simulink model "sim_discrete_planar_2dof"  (R2022b)
settings: Solver=VariableStepAuto  StartTime=0.0  StopTime=T_sim  FixedStep=param_global.Ta  RelTol=1e-3
InitFcn:
  | parameters_2dof;
undefined symbols (not defined in the file): coord_xaxis_len, coord_yaxis_len, coord_zaxis_len, fx, fy, fz, init_bus_param, param_global, param_path_set_points, param_robot, param_vis, path_coord_xaxis_len, path_coord_yaxis_len, path_coord_zaxis_len, path_param, q_0, q_0_p, tau_x, tau_y, tau_z, X, Y, Z
libraries: sm_lib x1241, nesl_utility x796
(part 33 of 34)

== system sim_discrete_planar_2dof/Visualization q2/static single path visualize spline (fast)/static single path visualize spline path4/Subsystem16 ==
  Spline : sm_lib/Curves and Surfaces/Spline  GraphicAmbientColor=[0.15 0.15 0.15 1.0]  GraphicDiffuseColor=path_coord_xaxis_color  DataPoints=[0 path_coord_xaxis_len; 0 0; 0 0]'  DataPointsUnits=cm  GraphicEmissiveColor=[0.0 0.0 0.0 1.0]  EndConditionType=Natural  MarkerShape=Sphere  MarkerSize=10  GraphicType=FromGeometry  GraphicOpacity=path_coord_xaxis_opacity  GraphicShininess=75  GraphicSpecularColor=[0.5 0.5 0.5 1.0]  GraphicVisPropType=SimpleVisualProperties
  Spline1 : sm_lib/Curves and Surfaces/Spline  GraphicAmbientColor=[0.15 0.15 0.15 1.0]  GraphicDiffuseColor=path_coord_yaxis_color  DataPoints=[0 0; 0 path_coord_yaxis_len; 0 0]'  DataPointsUnits=cm  GraphicEmissiveColor=[0.0 0.0 0.0 1.0]  EndConditionType=Natural  MarkerShape=Sphere  MarkerSize=10  GraphicType=FromGeometry  GraphicOpacity=path_coord_yaxis_opacity  GraphicShininess=75  GraphicSpecularColor=[0.5 0.5 0.5 1.0]  GraphicVisPropType=SimpleVisualProperties
  Spline2 : sm_lib/Curves and Surfaces/Spline  GraphicAmbientColor=[0.15 0.15 0.15 1.0]  GraphicDiffuseColor=path_coord_zaxis_color  DataPoints=[0 0; 0 0; 0 path_coord_zaxis_len]'  DataPointsUnits=cm  GraphicEmissiveColor=[0.0 0.0 0.0 1.0]  EndConditionType=Natural  MarkerShape=Sphere  MarkerSize=10  GraphicType=FromGeometry  GraphicOpacity=path_coord_zaxis_opacity  GraphicShininess=75  GraphicSpecularColor=[0.5 0.5 0.5 1.0]  GraphicVisPropType=SimpleVisualProperties
  path4_point : sm_lib/Curves and Surfaces/Point  GraphicType=Marker  MarkerShape=Sphere  MarkerSize=path_point_size  GraphicVisPropType=SimpleVisualProperties  GraphicDiffuseColor=path_point_color  GraphicOpacity=path_point_opacity  GraphicSpecularColor=[0.5 0.5 0.5 1.0]  GraphicAmbientColor=[0.15 0.15 0.15 1.0]  GraphicEmissiveColor=[0.0 0.0 0.0 1.0]  GraphicShininess=75
  world_iiwa_joint_OriginTransform4 : sm_lib/Frames and Transforms/Rigid Transform  TranslationMethod=Cartesian  TranslationStandardOffset=0  TranslationStandardOffsetUnits=m  TranslationStandardAxis=+Z  TranslationCartesianOffset=param_path_set_points.paths_set1(17, 1:3, 4)  TranslationCartesianOffsetUnits=m  TranslationROffset=0  TranslationROffsetUnits=m  TranslationZOffset=0  TranslationZOffsetUnits=m  TranslationThetaOffset=0  TranslationThetaOffsetUnits=deg  RotationMethod=RotationMatrix  RotationAngleUnits=deg  RotationStandardAxis=+Z  RotationAngle=0.0  RotationArbitraryAxis=[0 0 1]  FollAlignAxisA=+X  BaseAlignAxisA=+Y  FollAlignAxisB=+Y  BaseAlignAxisB=+Z  RotationSequenceAxes=BaseAxes  RotationSequence=XYZ  RotationSequenceAngles=[0, 0, 0]  RotationSequenceAnglesUnits=rad  RotationMatrix=eul2rotm(param_path_set_points.paths_set1(17, 4:6, 4), 'XYZ')  Quaternion=[1 0 0 0]
  coord : PMIOPort  Side=Left
  connections:
  coord/RConn1 -- world_iiwa_joint_OriginTransform4/LConn1
  path4_point/LConn1 -- world_iiwa_joint_OriginTransform4/RConn1 -- Spline/LConn1 -- Spline2/LConn1 -- Spline1/LConn1

== system sim_discrete_planar_2dof/Visualization q2/static single path visualize spline (fast)/static single path visualize spline path4/Subsystem17 ==
  Spline : sm_lib/Curves and Surfaces/Spline  GraphicAmbientColor=[0.15 0.15 0.15 1.0]  GraphicDiffuseColor=path_coord_xaxis_color  DataPoints=[0 path_coord_xaxis_len; 0 0; 0 0]'  DataPointsUnits=cm  GraphicEmissiveColor=[0.0 0.0 0.0 1.0]  EndConditionType=Natural  MarkerShape=Sphere  MarkerSize=10  GraphicType=FromGeometry  GraphicOpacity=path_coord_xaxis_opacity  GraphicShininess=75  GraphicSpecularColor=[0.5 0.5 0.5 1.0]  GraphicVisPropType=SimpleVisualProperties
  Spline1 : sm_lib/Curves and Surfaces/Spline  GraphicAmbientColor=[0.15 0.15 0.15 1.0]  GraphicDiffuseColor=path_coord_yaxis_color  DataPoints=[0 0; 0 path_coord_yaxis_len; 0 0]'  DataPointsUnits=cm  GraphicEmissiveColor=[0.0 0.0 0.0 1.0]  EndConditionType=Natural  MarkerShape=Sphere  MarkerSize=10  GraphicType=FromGeometry  GraphicOpacity=path_coord_yaxis_opacity  GraphicShininess=75  GraphicSpecularColor=[0.5 0.5 0.5 1.0]  GraphicVisPropType=SimpleVisualProperties
  Spline2 : sm_lib/Curves and Surfaces/Spline  GraphicAmbientColor=[0.15 0.15 0.15 1.0]  GraphicDiffuseColor=path_coord_zaxis_color  DataPoints=[0 0; 0 0; 0 path_coord_zaxis_len]'  DataPointsUnits=cm  GraphicEmissiveColor=[0.0 0.0 0.0 1.0]  EndConditionType=Natural  MarkerShape=Sphere  MarkerSize=10  GraphicType=FromGeometry  GraphicOpacity=path_coord_zaxis_opacity  GraphicShininess=75  GraphicSpecularColor=[0.5 0.5 0.5 1.0]  GraphicVisPropType=SimpleVisualProperties
  path4_point : sm_lib/Curves and Surfaces/Point  GraphicType=Marker  MarkerShape=Sphere  MarkerSize=path_point_size  GraphicVisPropType=SimpleVisualProperties  GraphicDiffuseColor=path_point_color  GraphicOpacity=path_point_opacity  GraphicSpecularColor=[0.5 0.5 0.5 1.0]  GraphicAmbientColor=[0.15 0.15 0.15 1.0]  GraphicEmissiveColor=[0.0 0.0 0.0 1.0]  GraphicShininess=75
  world_iiwa_joint_OriginTransform4 : sm_lib/Frames and Transforms/Rigid Transform  TranslationMethod=Cartesian  TranslationStandardOffset=0  TranslationStandardOffsetUnits=m  TranslationStandardAxis=+Z  TranslationCartesianOffset=param_path_set_points.paths_set1(18, 1:3, 4)  TranslationCartesianOffsetUnits=m  TranslationROffset=0  TranslationROffsetUnits=m  TranslationZOffset=0  TranslationZOffsetUnits=m  TranslationThetaOffset=0  TranslationThetaOffsetUnits=deg  RotationMethod=RotationMatrix  RotationAngleUnits=deg  RotationStandardAxis=+Z  RotationAngle=0.0  RotationArbitraryAxis=[0 0 1]  FollAlignAxisA=+X  BaseAlignAxisA=+Y  FollAlignAxisB=+Y  BaseAlignAxisB=+Z  RotationSequenceAxes=BaseAxes  RotationSequence=XYZ  RotationSequenceAngles=[0, 0, 0]  RotationSequenceAnglesUnits=rad  RotationMatrix=eul2rotm(param_path_set_points.paths_set1(18, 4:6, 4), 'XYZ')  Quaternion=[1 0 0 0]
  coord : PMIOPort  Side=Left
  connections:
  Spline1/LConn1 -- Spline2/LConn1 -- Spline/LConn1 -- world_iiwa_joint_OriginTransform4/RConn1 -- path4_point/LConn1
  coord/RConn1 -- world_iiwa_joint_OriginTransform4/LConn1

== system sim_discrete_planar_2dof/Visualization q2/static single path visualize spline (fast)/static single path visualize spline path4/Subsystem18 ==
  Spline : sm_lib/Curves and Surfaces/Spline  GraphicAmbientColor=[0.15 0.15 0.15 1.0]  GraphicDiffuseColor=path_coord_xaxis_color  DataPoints=[0 path_coord_xaxis_len; 0 0; 0 0]'  DataPointsUnits=cm  GraphicEmissiveColor=[0.0 0.0 0.0 1.0]  EndConditionType=Natural  MarkerShape=Sphere  MarkerSize=10  GraphicType=FromGeometry  GraphicOpacity=path_coord_xaxis_opacity  GraphicShininess=75  GraphicSpecularColor=[0.5 0.5 0.5 1.0]  GraphicVisPropType=SimpleVisualProperties
  Spline1 : sm_lib/Curves and Surfaces/Spline  GraphicAmbientColor=[0.15 0.15 0.15 1.0]  GraphicDiffuseColor=path_coord_yaxis_color  DataPoints=[0 0; 0 path_coord_yaxis_len; 0 0]'  DataPointsUnits=cm  GraphicEmissiveColor=[0.0 0.0 0.0 1.0]  EndConditionType=Natural  MarkerShape=Sphere  MarkerSize=10  GraphicType=FromGeometry  GraphicOpacity=path_coord_yaxis_opacity  GraphicShininess=75  GraphicSpecularColor=[0.5 0.5 0.5 1.0]  GraphicVisPropType=SimpleVisualProperties
  Spline2 : sm_lib/Curves and Surfaces/Spline  GraphicAmbientColor=[0.15 0.15 0.15 1.0]  GraphicDiffuseColor=path_coord_zaxis_color  DataPoints=[0 0; 0 0; 0 path_coord_zaxis_len]'  DataPointsUnits=cm  GraphicEmissiveColor=[0.0 0.0 0.0 1.0]  EndConditionType=Natural  MarkerShape=Sphere  MarkerSize=10  GraphicType=FromGeometry  GraphicOpacity=path_coord_zaxis_opacity  GraphicShininess=75  GraphicSpecularColor=[0.5 0.5 0.5 1.0]  GraphicVisPropType=SimpleVisualProperties
  path4_point : sm_lib/Curves and Surfaces/Point  GraphicType=Marker  MarkerShape=Sphere  MarkerSize=path_point_size  GraphicVisPropType=SimpleVisualProperties  GraphicDiffuseColor=path_point_color  GraphicOpacity=path_point_opacity  GraphicSpecularColor=[0.5 0.5 0.5 1.0]  GraphicAmbientColor=[0.15 0.15 0.15 1.0]  GraphicEmissiveColor=[0.0 0.0 0.0 1.0]  GraphicShininess=75
  world_iiwa_joint_OriginTransform4 : sm_lib/Frames and Transforms/Rigid Transform  TranslationMethod=Cartesian  TranslationStandardOffset=0  TranslationStandardOffsetUnits=m  TranslationStandardAxis=+Z  TranslationCartesianOffset=param_path_set_points.paths_set1(19, 1:3, 4)  TranslationCartesianOffsetUnits=m  TranslationROffset=0  TranslationROffsetUnits=m  TranslationZOffset=0  TranslationZOffsetUnits=m  TranslationThetaOffset=0  TranslationThetaOffsetUnits=deg  RotationMethod=RotationMatrix  RotationAngleUnits=deg  RotationStandardAxis=+Z  RotationAngle=0.0  RotationArbitraryAxis=[0 0 1]  FollAlignAxisA=+X  BaseAlignAxisA=+Y  FollAlignAxisB=+Y  BaseAlignAxisB=+Z  RotationSequenceAxes=BaseAxes  RotationSequence=XYZ  RotationSequenceAngles=[0, 0, 0]  RotationSequenceAnglesUnits=rad  RotationMatrix=eul2rotm(param_path_set_points.paths_set1(19, 4:6, 4), 'XYZ')  Quaternion=[1 0 0 0]
  coord : PMIOPort  Side=Left
  connections:
  Spline1/LConn1 -- Spline2/LConn1 -- Spline/LConn1 -- world_iiwa_joint_OriginTransform4/RConn1 -- path4_point/LConn1
  coord/RConn1 -- world_iiwa_joint_OriginTransform4/LConn1

== system sim_discrete_planar_2dof/Visualization q2/static single path visualize spline (fast)/static single path visualize spline path4/Subsystem19 ==
  Spline : sm_lib/Curves and Surfaces/Spline  GraphicAmbientColor=[0.15 0.15 0.15 1.0]  GraphicDiffuseColor=path_coord_xaxis_color  DataPoints=[0 path_coord_xaxis_len; 0 0; 0 0]'  DataPointsUnits=cm  GraphicEmissiveColor=[0.0 0.0 0.0 1.0]  EndConditionType=Natural  MarkerShape=Sphere  MarkerSize=10  GraphicType=FromGeometry  GraphicOpacity=path_coord_xaxis_opacity  GraphicShininess=75  GraphicSpecularColor=[0.5 0.5 0.5 1.0]  GraphicVisPropType=SimpleVisualProperties
  Spline1 : sm_lib/Curves and Surfaces/Spline  GraphicAmbientColor=[0.15 0.15 0.15 1.0]  GraphicDiffuseColor=path_coord_yaxis_color  DataPoints=[0 0; 0 path_coord_yaxis_len; 0 0]'  DataPointsUnits=cm  GraphicEmissiveColor=[0.0 0.0 0.0 1.0]  EndConditionType=Natural  MarkerShape=Sphere  MarkerSize=10  GraphicType=FromGeometry  GraphicOpacity=path_coord_yaxis_opacity  GraphicShininess=75  GraphicSpecularColor=[0.5 0.5 0.5 1.0]  GraphicVisPropType=SimpleVisualProperties
  Spline2 : sm_lib/Curves and Surfaces/Spline  GraphicAmbientColor=[0.15 0.15 0.15 1.0]  GraphicDiffuseColor=path_coord_zaxis_color  DataPoints=[0 0; 0 0; 0 path_coord_zaxis_len]'  DataPointsUnits=cm  GraphicEmissiveColor=[0.0 0.0 0.0 1.0]  EndConditionType=Natural  MarkerShape=Sphere  MarkerSize=10  GraphicType=FromGeometry  GraphicOpacity=path_coord_zaxis_opacity  GraphicShininess=75  GraphicSpecularColor=[0.5 0.5 0.5 1.0]  GraphicVisPropType=SimpleVisualProperties
  path4_point : sm_lib/Curves and Surfaces/Point  GraphicType=Marker  MarkerShape=Sphere  MarkerSize=path_point_size  GraphicVisPropType=SimpleVisualProperties  GraphicDiffuseColor=path_point_color  GraphicOpacity=path_point_opacity  GraphicSpecularColor=[0.5 0.5 0.5 1.0]  GraphicAmbientColor=[0.15 0.15 0.15 1.0]  GraphicEmissiveColor=[0.0 0.0 0.0 1.0]  GraphicShininess=75
  world_iiwa_joint_OriginTransform4 : sm_lib/Frames and Transforms/Rigid Transform  TranslationMethod=Cartesian  TranslationStandardOffset=0  TranslationStandardOffsetUnits=m  TranslationStandardAxis=+Z  TranslationCartesianOffset=param_path_set_points.paths_set1(20, 1:3, 4)  TranslationCartesianOffsetUnits=m  TranslationROffset=0  TranslationROffsetUnits=m  TranslationZOffset=0  TranslationZOffsetUnits=m  TranslationThetaOffset=0  TranslationThetaOffsetUnits=deg  RotationMethod=RotationMatrix  RotationAngleUnits=deg  RotationStandardAxis=+Z  RotationAngle=0.0  RotationArbitraryAxis=[0 0 1]  FollAlignAxisA=+X  BaseAlignAxisA=+Y  FollAlignAxisB=+Y  BaseAlignAxisB=+Z  RotationSequenceAxes=BaseAxes  RotationSequence=XYZ  RotationSequenceAngles=[0, 0, 0]  RotationSequenceAnglesUnits=rad  RotationMatrix=eul2rotm(param_path_set_points.paths_set1(20, 4:6, 4), 'XYZ')  Quaternion=[1 0 0 0]
  coord : PMIOPort  Side=Left
  connections:
  coord/RConn1 -- world_iiwa_joint_OriginTransform4/LConn1
  path4_point/LConn1 -- world_iiwa_joint_OriginTransform4/RConn1 -- Spline/LConn1 -- Spline2/LConn1 -- Spline1/LConn1

== system sim_discrete_planar_2dof/Visualization q2/static single path visualize spline (fast)/static single path visualize spline path4/Subsystem2 ==
  Spline : sm_lib/Curves and Surfaces/Spline  GraphicAmbientColor=[0.15 0.15 0.15 1.0]  GraphicDiffuseColor=path_coord_xaxis_color  DataPoints=[0 path_coord_xaxis_len; 0 0; 0 0]'  DataPointsUnits=cm  GraphicEmissiveColor=[0.0 0.0 0.0 1.0]  EndConditionType=Natural  MarkerShape=Sphere  MarkerSize=10  GraphicType=FromGeometry  GraphicOpacity=path_coord_xaxis_opacity  GraphicShininess=75  GraphicSpecularColor=[0.5 0.5 0.5 1.0]  GraphicVisPropType=SimpleVisualProperties
  Spline1 : sm_lib/Curves and Surfaces/Spline  GraphicAmbientColor=[0.15 0.15 0.15 1.0]  GraphicDiffuseColor=path_coord_yaxis_color  DataPoints=[0 0; 0 path_coord_yaxis_len; 0 0]'  DataPointsUnits=cm  GraphicEmissiveColor=[0.0 0.0 0.0 1.0]  EndConditionType=Natural  MarkerShape=Sphere  MarkerSize=10  GraphicType=FromGeometry  GraphicOpacity=path_coord_yaxis_opacity  GraphicShininess=75  GraphicSpecularColor=[0.5 0.5 0.5 1.0]  GraphicVisPropType=SimpleVisualProperties
  Spline2 : sm_lib/Curves and Surfaces/Spline  GraphicAmbientColor=[0.15 0.15 0.15 1.0]  GraphicDiffuseColor=path_coord_zaxis_color  DataPoints=[0 0; 0 0; 0 path_coord_zaxis_len]'  DataPointsUnits=cm  GraphicEmissiveColor=[0.0 0.0 0.0 1.0]  EndConditionType=Natural  MarkerShape=Sphere  MarkerSize=10  GraphicType=FromGeometry  GraphicOpacity=path_coord_zaxis_opacity  GraphicShininess=75  GraphicSpecularColor=[0.5 0.5 0.5 1.0]  GraphicVisPropType=SimpleVisualProperties
  path4_point : sm_lib/Curves and Surfaces/Point  GraphicType=Marker  MarkerShape=Sphere  MarkerSize=path_point_size  GraphicVisPropType=SimpleVisualProperties  GraphicDiffuseColor=path_point_color  GraphicOpacity=path_point_opacity  GraphicSpecularColor=[0.5 0.5 0.5 1.0]  GraphicAmbientColor=[0.15 0.15 0.15 1.0]  GraphicEmissiveColor=[0.0 0.0 0.0 1.0]  GraphicShininess=75
  world_iiwa_joint_OriginTransform4 : sm_lib/Frames and Transforms/Rigid Transform  TranslationMethod=Cartesian  TranslationStandardOffset=0  TranslationStandardOffsetUnits=m  TranslationStandardAxis=+Z  TranslationCartesianOffset=param_path_set_points.paths_set1(3, 1:3, 4)  TranslationCartesianOffsetUnits=m  TranslationROffset=0  TranslationROffsetUnits=m  TranslationZOffset=0  TranslationZOffsetUnits=m  TranslationThetaOffset=0  TranslationThetaOffsetUnits=deg  RotationMethod=RotationMatrix  RotationAngleUnits=deg  RotationStandardAxis=+Z  RotationAngle=0.0  RotationArbitraryAxis=[0 0 1]  FollAlignAxisA=+X  BaseAlignAxisA=+Y  FollAlignAxisB=+Y  BaseAlignAxisB=+Z  RotationSequenceAxes=BaseAxes  RotationSequence=XYZ  RotationSequenceAngles=[0, 0, 0]  RotationSequenceAnglesUnits=rad  RotationMatrix=eul2rotm(param_path_set_points.paths_set1(3, 4:6, 4), 'XYZ')  Quaternion=[1 0 0 0]
  coord : PMIOPort  Side=Left
  connections:
  coord/RConn1 -- world_iiwa_joint_OriginTransform4/LConn1
  path4_point/LConn1 -- world_iiwa_joint_OriginTransform4/RConn1 -- Spline/LConn1 -- Spline2/LConn1 -- Spline1/LConn1

== system sim_discrete_planar_2dof/Visualization q2/static single path visualize spline (fast)/static single path visualize spline path4/Subsystem3 ==
  Spline : sm_lib/Curves and Surfaces/Spline  GraphicAmbientColor=[0.15 0.15 0.15 1.0]  GraphicDiffuseColor=path_coord_xaxis_color  DataPoints=[0 path_coord_xaxis_len; 0 0; 0 0]'  DataPointsUnits=cm  GraphicEmissiveColor=[0.0 0.0 0.0 1.0]  EndConditionType=Natural  MarkerShape=Sphere  MarkerSize=10  GraphicType=FromGeometry  GraphicOpacity=path_coord_xaxis_opacity  GraphicShininess=75  GraphicSpecularColor=[0.5 0.5 0.5 1.0]  GraphicVisPropType=SimpleVisualProperties
  Spline1 : sm_lib/Curves and Surfaces/Spline  GraphicAmbientColor=[0.15 0.15 0.15 1.0]  GraphicDiffuseColor=path_coord_yaxis_color  DataPoints=[0 0; 0 path_coord_yaxis_len; 0 0]'  DataPointsUnits=cm  GraphicEmissiveColor=[0.0 0.0 0.0 1.0]  EndConditionType=Natural  MarkerShape=Sphere  MarkerSize=10  GraphicType=FromGeometry  GraphicOpacity=path_coord_yaxis_opacity  GraphicShininess=75  GraphicSpecularColor=[0.5 0.5 0.5 1.0]  GraphicVisPropType=SimpleVisualProperties
  Spline2 : sm_lib/Curves and Surfaces/Spline  GraphicAmbientColor=[0.15 0.15 0.15 1.0]  GraphicDiffuseColor=path_coord_zaxis_color  DataPoints=[0 0; 0 0; 0 path_coord_zaxis_len]'  DataPointsUnits=cm  GraphicEmissiveColor=[0.0 0.0 0.0 1.0]  EndConditionType=Natural  MarkerShape=Sphere  MarkerSize=10  GraphicType=FromGeometry  GraphicOpacity=path_coord_zaxis_opacity  GraphicShininess=75  GraphicSpecularColor=[0.5 0.5 0.5 1.0]  GraphicVisPropType=SimpleVisualProperties
  path4_point : sm_lib/Curves and Surfaces/Point  GraphicType=Marker  MarkerShape=Sphere  MarkerSize=path_point_size  GraphicVisPropType=SimpleVisualProperties  GraphicDiffuseColor=path_point_color  GraphicOpacity=path_point_opacity  GraphicSpecularColor=[0.5 0.5 0.5 1.0]  GraphicAmbientColor=[0.15 0.15 0.15 1.0]  GraphicEmissiveColor=[0.0 0.0 0.0 1.0]  GraphicShininess=75
  world_iiwa_joint_OriginTransform4 : sm_lib/Frames and Transforms/Rigid Transform  TranslationMethod=Cartesian  TranslationStandardOffset=0  TranslationStandardOffsetUnits=m  TranslationStandardAxis=+Z  TranslationCartesianOffset=param_path_set_points.paths_set1(4, 1:3, 4)  TranslationCartesianOffsetUnits=m  TranslationROffset=0  TranslationROffsetUnits=m  TranslationZOffset=0  TranslationZOffsetUnits=m  TranslationThetaOffset=0  TranslationThetaOffsetUnits=deg  RotationMethod=RotationMatrix  RotationAngleUnits=deg  RotationStandardAxis=+Z  RotationAngle=0.0  RotationArbitraryAxis=[0 0 1]  FollAlignAxisA=+X  BaseAlignAxisA=+Y  FollAlignAxisB=+Y  BaseAlignAxisB=+Z  RotationSequenceAxes=BaseAxes  RotationSequence=XYZ  RotationSequenceAngles=[0, 0, 0]  RotationSequenceAnglesUnits=rad  RotationMatrix=eul2rotm(param_path_set_points.paths_set1(4, 4:6, 4), 'XYZ')  Quaternion=[1 0 0 0]
  coord : PMIOPort  Side=Left
  connections:
  Spline1/LConn1 -- Spline2/LConn1 -- Spline/LConn1 -- world_iiwa_joint_OriginTransform4/RConn1 -- path4_point/LConn1
  coord/RConn1 -- world_iiwa_joint_OriginTransform4/LConn1

== system sim_discrete_planar_2dof/Visualization q2/static single path visualize spline (fast)/static single path visualize spline path4/Subsystem4 ==
  Spline : sm_lib/Curves and Surfaces/Spline  GraphicAmbientColor=[0.15 0.15 0.15 1.0]  GraphicDiffuseColor=path_coord_xaxis_color  DataPoints=[0 path_coord_xaxis_len; 0 0; 0 0]'  DataPointsUnits=cm  GraphicEmissiveColor=[0.0 0.0 0.0 1.0]  EndConditionType=Natural  MarkerShape=Sphere  MarkerSize=10  GraphicType=FromGeometry  GraphicOpacity=path_coord_xaxis_opacity  GraphicShininess=75  GraphicSpecularColor=[0.5 0.5 0.5 1.0]  GraphicVisPropType=SimpleVisualProperties
  Spline1 : sm_lib/Curves and Surfaces/Spline  GraphicAmbientColor=[0.15 0.15 0.15 1.0]  GraphicDiffuseColor=path_coord_yaxis_color  DataPoints=[0 0; 0 path_coord_yaxis_len; 0 0]'  DataPointsUnits=cm  GraphicEmissiveColor=[0.0 0.0 0.0 1.0]  EndConditionType=Natural  MarkerShape=Sphere  MarkerSize=10  GraphicType=FromGeometry  GraphicOpacity=path_coord_yaxis_opacity  GraphicShininess=75  GraphicSpecularColor=[0.5 0.5 0.5 1.0]  GraphicVisPropType=SimpleVisualProperties
  Spline2 : sm_lib/Curves and Surfaces/Spline  GraphicAmbientColor=[0.15 0.15 0.15 1.0]  GraphicDiffuseColor=path_coord_zaxis_color  DataPoints=[0 0; 0 0; 0 path_coord_zaxis_len]'  DataPointsUnits=cm  GraphicEmissiveColor=[0.0 0.0 0.0 1.0]  EndConditionType=Natural  MarkerShape=Sphere  MarkerSize=10  GraphicType=FromGeometry  GraphicOpacity=path_coord_zaxis_opacity  GraphicShininess=75  GraphicSpecularColor=[0.5 0.5 0.5 1.0]  GraphicVisPropType=SimpleVisualProperties
  path4_point : sm_lib/Curves and Surfaces/Point  GraphicType=Marker  MarkerShape=Sphere  MarkerSize=path_point_size  GraphicVisPropType=SimpleVisualProperties  GraphicDiffuseColor=path_point_color  GraphicOpacity=path_point_opacity  GraphicSpecularColor=[0.5 0.5 0.5 1.0]  GraphicAmbientColor=[0.15 0.15 0.15 1.0]  GraphicEmissiveColor=[0.0 0.0 0.0 1.0]  GraphicShininess=75
  world_iiwa_joint_OriginTransform4 : sm_lib/Frames and Transforms/Rigid Transform  TranslationMethod=Cartesian  TranslationStandardOffset=0  TranslationStandardOffsetUnits=m  TranslationStandardAxis=+Z  TranslationCartesianOffset=param_path_set_points.paths_set1(5, 1:3, 4)  TranslationCartesianOffsetUnits=m  TranslationROffset=0  TranslationROffsetUnits=m  TranslationZOffset=0  TranslationZOffsetUnits=m  TranslationThetaOffset=0  TranslationThetaOffsetUnits=deg  RotationMethod=RotationMatrix  RotationAngleUnits=deg  RotationStandardAxis=+Z  RotationAngle=0.0  RotationArbitraryAxis=[0 0 1]  FollAlignAxisA=+X  BaseAlignAxisA=+Y  FollAlignAxisB=+Y  BaseAlignAxisB=+Z  RotationSequenceAxes=BaseAxes  RotationSequence=XYZ  RotationSequenceAngles=[0, 0, 0]  RotationSequenceAnglesUnits=rad  RotationMatrix=eul2rotm(param_path_set_points.paths_set1(5, 4:6, 4), 'XYZ')  Quaternion=[1 0 0 0]
  coord : PMIOPort  Side=Left
  connections:
  coord/RConn1 -- world_iiwa_joint_OriginTransform4/LConn1
  path4_point/LConn1 -- world_iiwa_joint_OriginTransform4/RConn1 -- Spline/LConn1 -- Spline2/LConn1 -- Spline1/LConn1

== system sim_discrete_planar_2dof/Visualization q2/static single path visualize spline (fast)/static single path visualize spline path4/Subsystem5 ==
  Spline : sm_lib/Curves and Surfaces/Spline  GraphicAmbientColor=[0.15 0.15 0.15 1.0]  GraphicDiffuseColor=path_coord_xaxis_color  DataPoints=[0 path_coord_xaxis_len; 0 0; 0 0]'  DataPointsUnits=cm  GraphicEmissiveColor=[0.0 0.0 0.0 1.0]  EndConditionType=Natural  MarkerShape=Sphere  MarkerSize=10  GraphicType=FromGeometry  GraphicOpacity=path_coord_xaxis_opacity  GraphicShininess=75  GraphicSpecularColor=[0.5 0.5 0.5 1.0]  GraphicVisPropType=SimpleVisualProperties
  Spline1 : sm_lib/Curves and Surfaces/Spline  GraphicAmbientColor=[0.15 0.15 0.15 1.0]  GraphicDiffuseColor=path_coord_yaxis_color  DataPoints=[0 0; 0 path_coord_yaxis_len; 0 0]'  DataPointsUnits=cm  GraphicEmissiveColor=[0.0 0.0 0.0 1.0]  EndConditionType=Natural  MarkerShape=Sphere  MarkerSize=10  GraphicType=FromGeometry  GraphicOpacity=path_coord_yaxis_opacity  GraphicShininess=75  GraphicSpecularColor=[0.5 0.5 0.5 1.0]  GraphicVisPropType=SimpleVisualProperties
  Spline2 : sm_lib/Curves and Surfaces/Spline  GraphicAmbientColor=[0.15 0.15 0.15 1.0]  GraphicDiffuseColor=path_coord_zaxis_color  DataPoints=[0 0; 0 0; 0 path_coord_zaxis_len]'  DataPointsUnits=cm  GraphicEmissiveColor=[0.0 0.0 0.0 1.0]  EndConditionType=Natural  MarkerShape=Sphere  MarkerSize=10  GraphicType=FromGeometry  GraphicOpacity=path_coord_zaxis_opacity  GraphicShininess=75  GraphicSpecularColor=[0.5 0.5 0.5 1.0]  GraphicVisPropType=SimpleVisualProperties
  path4_point : sm_lib/Curves and Surfaces/Point  GraphicType=Marker  MarkerShape=Sphere  MarkerSize=path_point_size  GraphicVisPropType=SimpleVisualProperties  GraphicDiffuseColor=path_point_color  GraphicOpacity=path_point_opacity  GraphicSpecularColor=[0.5 0.5 0.5 1.0]  GraphicAmbientColor=[0.15 0.15 0.15 1.0]  GraphicEmissiveColor=[0.0 0.0 0.0 1.0]  GraphicShininess=75
  world_iiwa_joint_OriginTransform4 : sm_lib/Frames and Transforms/Rigid Transform  TranslationMethod=Cartesian  TranslationStandardOffset=0  TranslationStandardOffsetUnits=m  TranslationStandardAxis=+Z  TranslationCartesianOffset=param_path_set_points.paths_set1(6, 1:3, 4)  TranslationCartesianOffsetUnits=m  TranslationROffset=0  TranslationROffsetUnits=m  TranslationZOffset=0  TranslationZOffsetUnits=m  TranslationThetaOffset=0  TranslationThetaOffsetUnits=deg  RotationMethod=RotationMatrix  RotationAngleUnits=deg  RotationStandardAxis=+Z  RotationAngle=0.0  RotationArbitraryAxis=[0 0 1]  FollAlignAxisA=+X  BaseAlignAxisA=+Y  FollAlignAxisB=+Y  BaseAlignAxisB=+Z  RotationSequenceAxes=BaseAxes  RotationSequence=XYZ  RotationSequenceAngles=[0, 0, 0]  RotationSequenceAnglesUnits=rad  RotationMatrix=eul2rotm(param_path_set_points.paths_set1(6, 4:6, 4), 'XYZ')  Quaternion=[1 0 0 0]
  coord : PMIOPort  Side=Left
  connections:
  Spline1/LConn1 -- Spline2/LConn1 -- Spline/LConn1 -- world_iiwa_joint_OriginTransform4/RConn1 -- path4_point/LConn1
  coord/RConn1 -- world_iiwa_joint_OriginTransform4/LConn1

== system sim_discrete_planar_2dof/Visualization q2/static single path visualize spline (fast)/static single path visualize spline path4/Subsystem6 ==
  Spline : sm_lib/Curves and Surfaces/Spline  GraphicAmbientColor=[0.15 0.15 0.15 1.0]  GraphicDiffuseColor=path_coord_xaxis_color  DataPoints=[0 path_coord_xaxis_len; 0 0; 0 0]'  DataPointsUnits=cm  GraphicEmissiveColor=[0.0 0.0 0.0 1.0]  EndConditionType=Natural  MarkerShape=Sphere  MarkerSize=10  GraphicType=FromGeometry  GraphicOpacity=path_coord_xaxis_opacity  GraphicShininess=75  GraphicSpecularColor=[0.5 0.5 0.5 1.0]  GraphicVisPropType=SimpleVisualProperties
  Spline1 : sm_lib/Curves and Surfaces/Spline  GraphicAmbientColor=[0.15 0.15 0.15 1.0]  GraphicDiffuseColor=path_coord_yaxis_color  DataPoints=[0 0; 0 path_coord_yaxis_len; 0 0]'  DataPointsUnits=cm  GraphicEmissiveColor=[0.0 0.0 0.0 1.0]  EndConditionType=Natural  MarkerShape=Sphere  MarkerSize=10  GraphicType=FromGeometry  GraphicOpacity=path_coord_yaxis_opacity  GraphicShininess=75  GraphicSpecularColor=[0.5 0.5 0.5 1.0]  GraphicVisPropType=SimpleVisualProperties
  Spline2 : sm_lib/Curves and Surfaces/Spline  GraphicAmbientColor=[0.15 0.15 0.15 1.0]  GraphicDiffuseColor=path_coord_zaxis_color  DataPoints=[0 0; 0 0; 0 path_coord_zaxis_len]'  DataPointsUnits=cm  GraphicEmissiveColor=[0.0 0.0 0.0 1.0]  EndConditionType=Natural  MarkerShape=Sphere  MarkerSize=10  GraphicType=FromGeometry  GraphicOpacity=path_coord_zaxis_opacity  GraphicShininess=75  GraphicSpecularColor=[0.5 0.5 0.5 1.0]  GraphicVisPropType=SimpleVisualProperties
  path4_point : sm_lib/Curves and Surfaces/Point  GraphicType=Marker  MarkerShape=Sphere  MarkerSize=path_point_size  GraphicVisPropType=SimpleVisualProperties  GraphicDiffuseColor=path_point_color  GraphicOpacity=path_point_opacity  GraphicSpecularColor=[0.5 0.5 0.5 1.0]  GraphicAmbientColor=[0.15 0.15 0.15 1.0]  GraphicEmissiveColor=[0.0 0.0 0.0 1.0]  GraphicShininess=75
  world_iiwa_joint_OriginTransform4 : sm_lib/Frames and Transforms/Rigid Transform  TranslationMethod=Cartesian  TranslationStandardOffset=0  TranslationStandardOffsetUnits=m  TranslationStandardAxis=+Z  TranslationCartesianOffset=param_path_set_points.paths_set1(7, 1:3, 4)  TranslationCartesianOffsetUnits=m  TranslationROffset=0  TranslationROffsetUnits=m  TranslationZOffset=0  TranslationZOffsetUnits=m  TranslationThetaOffset=0  TranslationThetaOffsetUnits=deg  RotationMethod=RotationMatrix  RotationAngleUnits=deg  RotationStandardAxis=+Z  RotationAngle=0.0  RotationArbitraryAxis=[0 0 1]  FollAlignAxisA=+X  BaseAlignAxisA=+Y  FollAlignAxisB=+Y  BaseAlignAxisB=+Z  RotationSequenceAxes=BaseAxes  RotationSequence=XYZ  RotationSequenceAngles=[0, 0, 0]  RotationSequenceAnglesUnits=rad  RotationMatrix=eul2rotm(param_path_set_points.paths_set1(7, 4:6, 4), 'XYZ')  Quaternion=[1 0 0 0]
  coord : PMIOPort  Side=Left
  connections:
  Spline1/LConn1 -- Spline2/LConn1 -- Spline/LConn1 -- world_iiwa_joint_OriginTransform4/RConn1 -- path4_point/LConn1
  coord/RConn1 -- world_iiwa_joint_OriginTransform4/LConn1

== system sim_discrete_planar_2dof/Visualization q2/static single path visualize spline (fast)/static single path visualize spline path4/Subsystem7 ==
  Spline : sm_lib/Curves and Surfaces/Spline  GraphicAmbientColor=[0.15 0.15 0.15 1.0]  GraphicDiffuseColor=path_coord_xaxis_color  DataPoints=[0 path_coord_xaxis_len; 0 0; 0 0]'  DataPointsUnits=cm  GraphicEmissiveColor=[0.0 0.0 0.0 1.0]  EndConditionType=Natural  MarkerShape=Sphere  MarkerSize=10  GraphicType=FromGeometry  GraphicOpacity=path_coord_xaxis_opacity  GraphicShininess=75  GraphicSpecularColor=[0.5 0.5 0.5 1.0]  GraphicVisPropType=SimpleVisualProperties
  Spline1 : sm_lib/Curves and Surfaces/Spline  GraphicAmbientColor=[0.15 0.15 0.15 1.0]  GraphicDiffuseColor=path_coord_yaxis_color  DataPoints=[0 0; 0 path_coord_yaxis_len; 0 0]'  DataPointsUnits=cm  GraphicEmissiveColor=[0.0 0.0 0.0 1.0]  EndConditionType=Natural  MarkerShape=Sphere  MarkerSize=10  GraphicType=FromGeometry  GraphicOpacity=path_coord_yaxis_opacity  GraphicShininess=75  GraphicSpecularColor=[0.5 0.5 0.5 1.0]  GraphicVisPropType=SimpleVisualProperties
  Spline2 : sm_lib/Curves and Surfaces/Spline  GraphicAmbientColor=[0.15 0.15 0.15 1.0]  GraphicDiffuseColor=path_coord_zaxis_color  DataPoints=[0 0; 0 0; 0 path_coord_zaxis_len]'  DataPointsUnits=cm  GraphicEmissiveColor=[0.0 0.0 0.0 1.0]  EndConditionType=Natural  MarkerShape=Sphere  MarkerSize=10  GraphicType=FromGeometry  GraphicOpacity=path_coord_zaxis_opacity  GraphicShininess=75  GraphicSpecularColor=[0.5 0.5 0.5 1.0]  GraphicVisPropType=SimpleVisualProperties
  path4_point : sm_lib/Curves and Surfaces/Point  GraphicType=Marker  MarkerShape=Sphere  MarkerSize=path_point_size  GraphicVisPropType=SimpleVisualProperties  GraphicDiffuseColor=path_point_color  GraphicOpacity=path_point_opacity  GraphicSpecularColor=[0.5 0.5 0.5 1.0]  GraphicAmbientColor=[0.15 0.15 0.15 1.0]  GraphicEmissiveColor=[0.0 0.0 0.0 1.0]  GraphicShininess=75
  world_iiwa_joint_OriginTransform4 : sm_lib/Frames and Transforms/Rigid Transform  TranslationMethod=Cartesian  TranslationStandardOffset=0  TranslationStandardOffsetUnits=m  TranslationStandardAxis=+Z  TranslationCartesianOffset=param_path_set_points.paths_set1(8, 1:3, 4)  TranslationCartesianOffsetUnits=m  TranslationROffset=0  TranslationROffsetUnits=m  TranslationZOffset=0  TranslationZOffsetUnits=m  TranslationThetaOffset=0  TranslationThetaOffsetUnits=deg  RotationMethod=RotationMatrix  RotationAngleUnits=deg  RotationStandardAxis=+Z  RotationAngle=0.0  RotationArbitraryAxis=[0 0 1]  FollAlignAxisA=+X  BaseAlignAxisA=+Y  FollAlignAxisB=+Y  BaseAlignAxisB=+Z  RotationSequenceAxes=BaseAxes  RotationSequence=XYZ  RotationSequenceAngles=[0, 0, 0]  RotationSequenceAnglesUnits=rad  RotationMatrix=eul2rotm(param_path_set_points.paths_set1(8, 4:6, 4), 'XYZ')  Quaternion=[1 0 0 0]
  coord : PMIOPort  Side=Left
  connections:
  coord/RConn1 -- world_iiwa_joint_OriginTransform4/LConn1
  path4_point/LConn1 -- world_iiwa_joint_OriginTransform4/RConn1 -- Spline/LConn1 -- Spline2/LConn1 -- Spline1/LConn1

== system sim_discrete_planar_2dof/Visualization q2/static single path visualize spline (fast)/static single path visualize spline path4/Subsystem8 ==
  Spline : sm_lib/Curves and Surfaces/Spline  GraphicAmbientColor=[0.15 0.15 0.15 1.0]  GraphicDiffuseColor=path_coord_xaxis_color  DataPoints=[0 path_coord_xaxis_len; 0 0; 0 0]'  DataPointsUnits=cm  GraphicEmissiveColor=[0.0 0.0 0.0 1.0]  EndConditionType=Natural  MarkerShape=Sphere  MarkerSize=10  GraphicType=FromGeometry  GraphicOpacity=path_coord_xaxis_opacity  GraphicShininess=75  GraphicSpecularColor=[0.5 0.5 0.5 1.0]  GraphicVisPropType=SimpleVisualProperties
  Spline1 : sm_lib/Curves and Surfaces/Spline  GraphicAmbientColor=[0.15 0.15 0.15 1.0]  GraphicDiffuseColor=path_coord_yaxis_color  DataPoints=[0 0; 0 path_coord_yaxis_len; 0 0]'  DataPointsUnits=cm  GraphicEmissiveColor=[0.0 0.0 0.0 1.0]  EndConditionType=Natural  MarkerShape=Sphere  MarkerSize=10  GraphicType=FromGeometry  GraphicOpacity=path_coord_yaxis_opacity  GraphicShininess=75  GraphicSpecularColor=[0.5 0.5 0.5 1.0]  GraphicVisPropType=SimpleVisualProperties
  Spline2 : sm_lib/Curves and Surfaces/Spline  GraphicAmbientColor=[0.15 0.15 0.15 1.0]  GraphicDiffuseColor=path_coord_zaxis_color  DataPoints=[0 0; 0 0; 0 path_coord_zaxis_len]'  DataPointsUnits=cm  GraphicEmissiveColor=[0.0 0.0 0.0 1.0]  EndConditionType=Natural  MarkerShape=Sphere  MarkerSize=10  GraphicType=FromGeometry  GraphicOpacity=path_coord_zaxis_opacity  GraphicShininess=75  GraphicSpecularColor=[0.5 0.5 0.5 1.0]  GraphicVisPropType=SimpleVisualProperties
  path4_point : sm_lib/Curves and Surfaces/Point  GraphicType=Marker  MarkerShape=Sphere  MarkerSize=path_point_size  GraphicVisPropType=SimpleVisualProperties  GraphicDiffuseColor=path_point_color  GraphicOpacity=path_point_opacity  GraphicSpecularColor=[0.5 0.5 0.5 1.0]  GraphicAmbientColor=[0.15 0.15 0.15 1.0]  GraphicEmissiveColor=[0.0 0.0 0.0 1.0]  GraphicShininess=75
  world_iiwa_joint_OriginTransform4 : sm_lib/Frames and Transforms/Rigid Transform  TranslationMethod=Cartesian  TranslationStandardOffset=0  TranslationStandardOffsetUnits=m  TranslationStandardAxis=+Z  TranslationCartesianOffset=param_path_set_points.paths_set1(9, 1:3, 4)  TranslationCartesianOffsetUnits=m  TranslationROffset=0  TranslationROffsetUnits=m  TranslationZOffset=0  TranslationZOffsetUnits=m  TranslationThetaOffset=0  TranslationThetaOffsetUnits=deg  RotationMethod=RotationMatrix  RotationAngleUnits=deg  RotationStandardAxis=+Z  RotationAngle=0.0  RotationArbitraryAxis=[0 0 1]  FollAlignAxisA=+X  BaseAlignAxisA=+Y  FollAlignAxisB=+Y  BaseAlignAxisB=+Z  RotationSequenceAxes=BaseAxes  RotationSequence=XYZ  RotationSequenceAngles=[0, 0, 0]  RotationSequenceAnglesUnits=rad  RotationMatrix=eul2rotm(param_path_set_points.paths_set1(9, 4:6, 4), 'XYZ')  Quaternion=[1 0 0 0]
  coord : PMIOPort  Side=Left
  connections:
  coord/RConn1 -- world_iiwa_joint_OriginTransform4/LConn1
  path4_point/LConn1 -- world_iiwa_joint_OriginTransform4/RConn1 -- Spline/LConn1 -- Spline2/LConn1 -- Spline1/LConn1

== system sim_discrete_planar_2dof/Visualization q2/static single path visualize spline (fast)/static single path visualize spline path4/Subsystem9 ==
  Spline : sm_lib/Curves and Surfaces/Spline  GraphicAmbientColor=[0.15 0.15 0.15 1.0]  GraphicDiffuseColor=path_coord_xaxis_color  DataPoints=[0 path_coord_xaxis_len; 0 0; 0 0]'  DataPointsUnits=cm  GraphicEmissiveColor=[0.0 0.0 0.0 1.0]  EndConditionType=Natural  MarkerShape=Sphere  MarkerSize=10  GraphicType=FromGeometry  GraphicOpacity=path_coord_xaxis_opacity  GraphicShininess=75  GraphicSpecularColor=[0.5 0.5 0.5 1.0]  GraphicVisPropType=SimpleVisualProperties
  Spline1 : sm_lib/Curves and Surfaces/Spline  GraphicAmbientColor=[0.15 0.15 0.15 1.0]  GraphicDiffuseColor=path_coord_yaxis_color  DataPoints=[0 0; 0 path_coord_yaxis_len; 0 0]'  DataPointsUnits=cm  GraphicEmissiveColor=[0.0 0.0 0.0 1.0]  EndConditionType=Natural  MarkerShape=Sphere  MarkerSize=10  GraphicType=FromGeometry  GraphicOpacity=path_coord_yaxis_opacity  GraphicShininess=75  GraphicSpecularColor=[0.5 0.5 0.5 1.0]  GraphicVisPropType=SimpleVisualProperties
  Spline2 : sm_lib/Curves and Surfaces/Spline  GraphicAmbientColor=[0.15 0.15 0.15 1.0]  GraphicDiffuseColor=path_coord_zaxis_color  DataPoints=[0 0; 0 0; 0 path_coord_zaxis_len]'  DataPointsUnits=cm  GraphicEmissiveColor=[0.0 0.0 0.0 1.0]  EndConditionType=Natural  MarkerShape=Sphere  MarkerSize=10  GraphicType=FromGeometry  GraphicOpacity=path_coord_zaxis_opacity  GraphicShininess=75  GraphicSpecularColor=[0.5 0.5 0.5 1.0]  GraphicVisPropType=SimpleVisualProperties
  path4_point : sm_lib/Curves and Surfaces/Point  GraphicType=Marker  MarkerShape=Sphere  MarkerSize=path_point_size  GraphicVisPropType=SimpleVisualProperties  GraphicDiffuseColor=path_point_color  GraphicOpacity=path_point_opacity  GraphicSpecularColor=[0.5 0.5 0.5 1.0]  GraphicAmbientColor=[0.15 0.15 0.15 1.0]  GraphicEmissiveColor=[0.0 0.0 0.0 1.0]  GraphicShininess=75
  world_iiwa_joint_OriginTransform4 : sm_lib/Frames and Transforms/Rigid Transform  TranslationMethod=Cartesian  TranslationStandardOffset=0  TranslationStandardOffsetUnits=m  TranslationStandardAxis=+Z  TranslationCartesianOffset=param_path_set_points.paths_set1(10, 1:3, 4)  TranslationCartesianOffsetUnits=m  TranslationROffset=0  TranslationROffsetUnits=m  TranslationZOffset=0  TranslationZOffsetUnits=m  TranslationThetaOffset=0  TranslationThetaOffsetUnits=deg  RotationMethod=RotationMatrix  RotationAngleUnits=deg  RotationStandardAxis=+Z  RotationAngle=0.0  RotationArbitraryAxis=[0 0 1]  FollAlignAxisA=+X  BaseAlignAxisA=+Y  FollAlignAxisB=+Y  BaseAlignAxisB=+Z  RotationSequenceAxes=BaseAxes  RotationSequence=XYZ  RotationSequenceAngles=[0, 0, 0]  RotationSequenceAnglesUnits=rad  RotationMatrix=eul2rotm(param_path_set_points.paths_set1(10, 4:6, 4), 'XYZ')  Quaternion=[1 0 0 0]
  coord : PMIOPort  Side=Left
  connections:
  Spline1/LConn1 -- Spline2/LConn1 -- Spline/LConn1 -- world_iiwa_joint_OriginTransform4/RConn1 -- path4_point/LConn1
  coord/RConn1 -- world_iiwa_joint_OriginTransform4/LConn1

== matlab function sim_discrete_planar_2dof/Simulation models/controller/CT Controller Subsystem/CT controller ==
  settings: updateMethod=DISCRETE  sampleTime=param_global.Ta
  data: tau output; robot_model input Bus: robot_model; x_d input Bus: x_d; ct_ctrl_param parameter; debug_data output Bus: debug_data_bus
function [tau, debug_data] = fcn(robot_model, x_d, ct_ctrl_param)

% Parameters
%q = robot_model.q;      % generalized coordinates
q_p = robot_model.q_p;  % time derivative of generalized coordinates
q_pp = robot_model.q_pp;
M = robot_model.M;      % inertia matrix
C = robot_model.C;      % coriolis matrix
g = robot_model.g;      % gravitational forces
J = robot_model.J;      % geometric jacobian to end-effector
J_p = robot_model.J_p;  % time derivative of geometric jacobian to end-effector
H = robot_model.H;

J_v   = J(1:2, 1:2);
J_v_p = J_p(1:2, 1:2);

% Current Trajectory
p    = H(1:2,4);
p_p  = J_v*q_p;
p_pp = J_v*q_pp + J_v_p*q_p;

% Desired Trajectory
p_d    = x_d.p_d(1:2);
p_d_p  = x_d.p_d_p(1:2);
p_d_pp = x_d.p_d_pp(1:2);

% Control parameters
% kartesisch
Kp1 = ct_ctrl_param.Kp1;
Kd1 = ct_ctrl_param.Kd1;

% CT control law
%% Cartesian Endeffector Pose
%x_err = [ p- x_d.p_d; q_err(2:4)'];   % Error as quaternion
%x_err_p = [p_p - x_d.p_d_p; omega_e - x_d.omega_d];
%v = J\(x_d_pp  - Kd1*x_err_p - Kp1*x_err - J_p*q_p);
%v = inv(J(1:2,1:2))*(x_d_pp(1:2)  - Kd1(1:2,1:2)*x_err_p(1:2) - Kp1(1:2,1:2)*x_err(1:2) - J_p(1:2,1:2)*q_p);
sing_method = ct_ctrl_param.mode;

if(sing_method == 0)
    v = J_v\(p_d_pp - Kd1*(p_p - p_d_p) - Kp1*(p - p_d) - J_v_p*q_p);
elseif(sing_method == 1) % add +k*E
    k = ct_ctrl_param.k;
    J_pinv = (J_v'*J_v + k*eye(2))\J_v';
    v = J_pinv*(p_d_pp - Kd1*(p_p - p_d_p) - Kp1*(p - p_d) - J_v_p*q_p);
elseif(sing_method == 2) % add +lambda*E
    w_bar_N = ct_ctrl_param.w_bar_N;
    w_E = ct_ctrl_param.w_E;

    W_E = w_E.*diag([1 1]);
    e = (p - p_d);
    E_k = 1/2*e' * W_E * e;
    W_N = diag(E_k + w_bar_N);

    [U, S, V] = svd(sqrt(W_E)*J_v);

    %H_k = V*(S^2 + W_N)*V'; % geht nur wenn alle w_n gleich sind
    H_k = V*(S^2)*V' + W_N;
    J_pinv = H_k\J_v';
    v = J_pinv*(p_d_pp - Kd1*(p_p - p_d_p) - Kp1*(p - p_d) - J_v_p*q_p);

    %J_pinv = (J_v'*W_E*J_v + W_N)\J_v';
    %v = J_pinv*(p_d_pp - Kd1*(p_p - p_d_p) - Kp1*(p - p_d) - J_v_p*q_p);
    
    %J_new = (J_v'*W_E*J_v + W_N);
    %v = J_new\(p_d_pp - Kd1*(p_p - p_d_p) - Kp1*(p - p_d) - J_v_p*q_p);
else % remove small singular values
    % Perform SVD
    [U, S, V] = svd(J_v);
    
    % Set a threshold for small singular values
    epsilon = ct_ctrl_param.eps; % Adjust epsilon as needed
    
    % Threshold small singular values in S
    S_new = diag(max(diag(S), epsilon));  % Creates a diagonal matrix with thresholded values
    
    % Reconstruct the matrix with modified singular values
    J_new = U * S_new * V';
    v = J_new\(p_d_pp - Kd1*(p_p - p_d_p) - Kp1*(p - p_d) - J_v_p*q_p);
end

tau = M*v + C*q_p + g;

%% DEBUG
debug_data.p    = p;
debug_data.p_p  = p_p;
debug_data.p_pp = p_pp;
debug_data.rot_x_err = 0;
debug_data.rot_y_err = 0;
debug_data.rot_z_err = 0;

== matlab function sim_discrete_planar_2dof/Debug Subsystem/comp vel and acc ==
  settings: updateMethod=CONTINUOUS  sampleTime=inf
  data: robot_model input Bus: robot_model; p output; param_robot parameter; x_d_trj input Bus: x_d; tau input; p_d output; p_p output; p_d_p output; p_pp output; p_d_pp output
function [p, p_d, p_p, p_d_p, p_pp, p_d_pp]  = fcn(robot_model, param_robot, x_d_trj, tau)

q = robot_model.q;
q_p = robot_model.q_p;

%%
M=inertia_matrix(q,param_robot);                          % rigid body inertia matrix
C=coriolis_matrix(q,q_p,param_robot);                     % coriolis matrix
g=gravitational_forces(q,param_robot);                    % gravitational forces

% Equations of motion
%tau_lin = [Mrr,Mrq]*q_d_pp + [Crr,Crq]*q_p + g(1:2);
%q_pp = M\([tau_lin;tau_iiwa]-C*q_p-g);

q_pp = M\(tau -C*q_p - g);

%%

%q_pp = robot_model.q_pp;

H = hom_transform_endeffector(q, param_robot);
J = robot_model.J;
J_p = robot_model.J_p;

p = H(1:2, 4);
p_p = J(1:2,:)*q_p;
p_pp = J(1:2,:)*q_pp + J_p(1:2,:)*q_p;

p_d = x_d_trj.p_d(1:2);
p_d_p = x_d_trj.p_d_p(1:2);
p_d_pp = x_d_trj.p_d_pp(1:2);
